simulink library "inp"  (Simulink 8.2)
settings: Solver=ode45  SolverMode=SingleTasking  StartTime=0.0  StopTime=10.0  RelTol=1e-3
undefined symbols (not defined in the file or in its folder): C_TICK_TIME_S, ENUM_FAULTSTATE_FAIL, ENUM_FAULTSTATE_INDETERMINATE, ENUM_FAULTSTATE_PASS, ENUM_KEYSWITCH_ON, FALSE, KINP_ABS1CANCommuOk_flg_ovrdflg, KINP_ABS1CANCommuOk_flg_ovrdval, KINP_ABS1CANPeriodTime_s, KINP_ABS1CANRolCntOk_flg_ovrdflg, KINP_ABS1CANRolCntOk_flg_ovrdval, KINP_ABS2CANChkSumOk_flg_ovrdflg, KINP_ABS2CANChkSumOk_flg_ovrdval, KINP_ABS2CANCommuOk_flg_ovrdflg, KINP_ABS2CANCommuOk_flg_ovrdval, KINP_ABS2CANPeriodTime_s, KINP_ABS2CANRolCntOk_flg_ovrdflg, KINP_ABS2CANRolCntOk_flg_ovrdval, KINP_ABS3CANCommuOk_flg_ovrdflg, KINP_ABS3CANCommuOk_flg_ovrdval, KINP_ABS4CANCommuOk_flg_ovrdflg, KINP_ABS4CANCommuOk_flg_ovrdval, KINP_ABSActive_flg_defval, KINP_ABSActive_flg_invalid, KINP_ABSActive_flg_ovrdflg, KINP_ABSActive_flg_ovrdval, KINP_ABSActive_flg_setvalid, KINP_ABSActive_flg_srvcflg, KINP_ABSActive_flg_srvcval, KINP_ABSCANAlive_flg_ovrdflg, KINP_ABSCANAlive_flg_ovrdval, KINP_ABSCANCommuOk_flg_ovrdflg, KINP_ABSCANCommuOk_flg_ovrdval, KINP_ABSCANValidOk_flg_ovrdflg, KINP_ABSCANValidOk_flg_ovrdval, KINP_ABSFault_flg_defval, KINP_ABSFault_flg_invalid, KINP_ABSFault_flg_ovrdflg, KINP_ABSFault_flg_ovrdval, KINP_ABSFault_flg_setvalid, KINP_ABSFault_flg_srvcflg, KINP_ABSFault_flg_srvcval, KINP_AC1CANCommuOk_flg_ovrdflg, KINP_AC1CANCommuOk_flg_ovrdval, KINP_ACCANAlive_flg_ovrdflg, KINP_ACCANAlive_flg_ovrdval, KINP_ACCANCommuOk_flg_ovrdflg, KINP_ACCANCommuOk_flg_ovrdval, KINP_ACCANValidOk_flg_ovrdflg, KINP_ACCANValidOk_flg_ovrdval, KINP_ACCCancelH_flg_defval, KINP_ACCCancelH_flg_invalid, KINP_ACCCancelH_flg_ovrdflg, KINP_ACCCancelH_flg_ovrdval, KINP_ACCCancelH_flg_setvalid, KINP_ACCCancelH_flg_srvcflg, KINP_ACCCancelH_flg_srvcval, KINP_AccelPedalPosn_pct_defval, KINP_AccelPedalPosn_pct_gain, KINP_AccelPedalPosn_pct_invalid ... (+1866)
libraries: inp x192, hcu_lib x34, simulink x33
(part 10 of 21)

== system inp/INP/EPM_signals/Cam_Obstacle_3 ==
  BHAL_outputs : Inport
  CANvalid_bus : Inport  Port=2
  Bus Creator : BusCreator  Inputs=40
  CANVBusSel{41..50} (10 alike) : BusSelector  OutputSignals=VINP_CANMsgValidEPM_flg.VINP_CANMsgValidEPM12_flg
  CANVBusSel{51..55} (5 alike) : BusSelector  OutputSignals=VINP_CANMsgValidEPM_flg.VINP_CANMsgValidEPM13_flg
  CANVBusSel{56..60} (5 alike) : BusSelector  OutputSignals=VINP_CANMsgValidEPM_flg.VINP_CANMsgValidEPM14_flg
  Constant{41..60} (20 alike) : Constant  Value=TRUE
  Conv{41,45..49,53..54} (8 alike) : DataTypeConversion  OutDataTypeStr=uint8
  Conv{42..44,51..52,56..59} (9 alike) : DataTypeConversion  OutDataTypeStr=single
  Conv50 : DataTypeConversion  OutDataTypeStr=boolean
  Conv55 : DataTypeConversion  OutDataTypeStr=boolean
  Conv60 : DataTypeConversion  OutDataTypeStr=boolean
  HalBusSel41 : BusSelector  OutputSignals=VHAL_CamObs3ID_num
  HalBusSel42 : BusSelector  OutputSignals=VHAL_CamObs3PosX_m
  HalBusSel43 : BusSelector  OutputSignals=VHAL_CamObs3PosY_m
  HalBusSel44 : BusSelector  OutputSignals=VHAL_CamObs3VelX_mps
  HalBusSel45 : BusSelector  OutputSignals=VHAL_CamObs3Type_enum
  HalBusSel46 : BusSelector  OutputSignals=VHAL_CamObs3Status_enum
  HalBusSel47 : BusSelector  OutputSignals=VHAL_CamObs3Valid_enum
  HalBusSel48 : BusSelector  OutputSignals=VHAL_CamObs3MoveInO_enum
  HalBusSel49 : BusSelector  OutputSignals=VHAL_CamObs3Blinker_enum
  HalBusSel50 : BusSelector  OutputSignals=VHAL_CamObs3BrakeLight_flg
  HalBusSel51 : BusSelector  OutputSignals=VHAL_CamObs3Length_m
  HalBusSel52 : BusSelector  OutputSignals=VHAL_CamObs3Width_m
  HalBusSel53 : BusSelector  OutputSignals=VHAL_CamObs3Age_cnt
  HalBusSel54 : BusSelector  OutputSignals=VHAL_CamObs3Lane_enum
  HalBusSel55 : BusSelector  OutputSignals=VHAL_CamObs3CIPV_flg
  HalBusSel56 : BusSelector  OutputSignals=VHAL_CamObs3VelY_mps
  HalBusSel57 : BusSelector  OutputSignals=VHAL_CamObs3AccX_mps2
  HalBusSel58 : BusSelector  OutputSignals=VHAL_CamObs3Ang_deg
  HalBusSel59 : BusSelector  OutputSignals=VHAL_CamObs3AngRate_degps
  HalBusSel60 : BusSelector  OutputSignals=VHAL_CamObs3Replaced_flg
  Term{41..60} (20 alike) : Terminator
  Cam_Obstacle_3 : Outport
  wires:
  CANVBusSel55/1 -> Term55/1  "<VINP_CANMsgValidEPM13_flg>"
  HalBusSel41/1 -> Conv41/1  "<VHAL_CamObs3ID_num>"
  [BHAL_outputs] -> HalBusSel41/1
  [CANvalid_bus] -> CANVBusSel41/1
  Constant41/1 -> Bus Creator/2  "VINP_CamObs3IDValid_flg_InHAL"
  Conv41/1 -> Bus Creator/1  "VINP_CamObs3ID_num_InHAL"
  CANVBusSel41/1 -> Term41/1  "<VINP_CANMsgValidEPM12_flg>"
  [CANvalid_bus] -> CANVBusSel42/1
  [BHAL_outputs] -> HalBusSel42/1
  HalBusSel42/1 -> Conv42/1  "<VHAL_CamObs3PosX_m>"
  Constant42/1 -> Bus Creator/4  "VINP_CamObs3PosXValid_flg_InHAL"
  Conv42/1 -> Bus Creator/3  "VINP_CamObs3PosX_m_InHAL"
  CANVBusSel42/1 -> Term42/1  "<VINP_CANMsgValidEPM12_flg>"
  HalBusSel43/1 -> Conv43/1  "<VHAL_CamObs3PosY_m>"
  [BHAL_outputs] -> HalBusSel43/1
  [CANvalid_bus] -> CANVBusSel43/1
  Constant43/1 -> Bus Creator/6  "VINP_CamObs3PosYValid_flg_InHAL"
  Conv43/1 -> Bus Creator/5  "VINP_CamObs3PosY_m_InHAL"
  CANVBusSel43/1 -> Term43/1  "<VINP_CANMsgValidEPM12_flg>"
  HalBusSel44/1 -> Conv44/1  "<VHAL_CamObs3VelX_mps>"
  [BHAL_outputs] -> HalBusSel44/1
  [CANvalid_bus] -> CANVBusSel44/1
  Constant44/1 -> Bus Creator/8  "VINP_CamObs3VelXValid_flg_InHAL"
  Conv44/1 -> Bus Creator/7  "VINP_CamObs3VelX_mps_InHAL"
  CANVBusSel44/1 -> Term44/1  "<VINP_CANMsgValidEPM12_flg>"
  HalBusSel45/1 -> Conv45/1  "<VHAL_CamObs3Type_enum>"
  [BHAL_outputs] -> HalBusSel45/1
  [CANvalid_bus] -> CANVBusSel45/1
  Constant45/1 -> Bus Creator/10  "VINP_CamObs3TypeValid_flg_InHAL"
  Conv45/1 -> Bus Creator/9  "VINP_CamObs3Type_enum_InHAL"
  CANVBusSel45/1 -> Term45/1  "<VINP_CANMsgValidEPM12_flg>"
  [BHAL_outputs] -> HalBusSel46/1
  HalBusSel46/1 -> Conv46/1  "<VHAL_CamObs3Status_enum>"
  Conv46/1 -> Bus Creator/11  "VINP_CamObs3Status_enum_InHAL"
  Constant46/1 -> Bus Creator/12  "VINP_CamObs3StatusValid_flg_InHAL"
  [CANvalid_bus] -> CANVBusSel46/1
  CANVBusSel46/1 -> Term46/1  "<VINP_CANMsgValidEPM12_flg>"
  [BHAL_outputs] -> HalBusSel47/1
  HalBusSel47/1 -> Conv47/1  "<VHAL_CamObs3Valid_enum>"
  [CANvalid_bus] -> CANVBusSel47/1
  Constant47/1 -> Bus Creator/14  "VINP_CamObs3ValidValid_flg_InHAL"
  Conv47/1 -> Bus Creator/13  "VINP_CamObs3Valid_enum_InHAL"
  CANVBusSel47/1 -> Term47/1  "<VINP_CANMsgValidEPM12_flg>"
  [BHAL_outputs] -> HalBusSel48/1
  HalBusSel48/1 -> Conv48/1  "<VHAL_CamObs3MoveInO_enum>"
  Conv48/1 -> Bus Creator/15  "VINP_CamObs3MoveInO_enum_InHAL"
  Constant48/1 -> Bus Creator/16  "VINP_CamObs3MoveInOValid_flg_InHAL"
  [CANvalid_bus] -> CANVBusSel48/1
  CANVBusSel48/1 -> Term48/1  "<VINP_CANMsgValidEPM12_flg>"
  [BHAL_outputs] -> HalBusSel49/1
  HalBusSel49/1 -> Conv49/1  "<VHAL_CamObs3Blinker_enum>"
  Conv49/1 -> Bus Creator/17  "VINP_CamObs3Blinker_enum_InHAL"
  Constant49/1 -> Bus Creator/18  "VINP_CamObs3BlinkerValid_flg_InHAL"
  [CANvalid_bus] -> CANVBusSel49/1
  CANVBusSel49/1 -> Term49/1  "<VINP_CANMsgValidEPM12_flg>"
  [BHAL_outputs] -> HalBusSel50/1
  HalBusSel50/1 -> Conv50/1  "<VHAL_CamObs3BrakeLight_flg>"
  Conv50/1 -> Bus Creator/19  "VINP_CamObs3BrakeLight_flg_InHAL"
  Constant50/1 -> Bus Creator/20  "VINP_CamObs3BrakeLightValid_flg_InHAL"
  [CANvalid_bus] -> CANVBusSel50/1
  CANVBusSel50/1 -> Term50/1  "<VINP_CANMsgValidEPM12_flg>"
  [BHAL_outputs] -> HalBusSel51/1
  [CANvalid_bus] -> CANVBusSel51/1
  HalBusSel51/1 -> Conv51/1  "<VHAL_CamObs3Length_m>"
  Constant51/1 -> Bus Creator/22  "VINP_CamObs3LengthValid_flg_InHAL"
  Conv51/1 -> Bus Creator/21  "VINP_CamObs3Length_m_InHAL"
  CANVBusSel51/1 -> Term51/1  "<VINP_CANMsgValidEPM13_flg>"
  [BHAL_outputs] -> HalBusSel52/1
  [CANvalid_bus] -> CANVBusSel52/1
  HalBusSel52/1 -> Conv52/1  "<VHAL_CamObs3Width_m>"
  Constant52/1 -> Bus Creator/24  "VINP_CamObs3WidthValid_flg_InHAL"
  Conv52/1 -> Bus Creator/23  "VINP_CamObs3Width_m_InHAL"
  CANVBusSel52/1 -> Term52/1  "<VINP_CANMsgValidEPM13_flg>"
  [BHAL_outputs] -> HalBusSel53/1
  [CANvalid_bus] -> CANVBusSel53/1
  HalBusSel53/1 -> Conv53/1  "<VHAL_CamObs3Age_cnt>"
  Constant53/1 -> Bus Creator/26  "VINP_CamObs3AgeValid_flg_InHAL"
  Conv53/1 -> Bus Creator/25  "VINP_CamObs3Age_cnt_InHAL"
  CANVBusSel53/1 -> Term53/1  "<VINP_CANMsgValidEPM13_flg>"
  HalBusSel54/1 -> Conv54/1  "<VHAL_CamObs3Lane_enum>"
  [BHAL_outputs] -> HalBusSel54/1
  [CANvalid_bus] -> CANVBusSel54/1
  Constant54/1 -> Bus Creator/28  "VINP_CamObs3LaneValid_flg_InHAL"
  Conv54/1 -> Bus Creator/27  "VINP_CamObs3Lane_enum_InHAL"
  CANVBusSel54/1 -> Term54/1  "<VINP_CANMsgValidEPM13_flg>"
  HalBusSel55/1 -> Conv55/1  "<VHAL_CamObs3CIPV_flg>"
  [BHAL_outputs] -> HalBusSel55/1
  [CANvalid_bus] -> CANVBusSel55/1
  Constant55/1 -> Bus Creator/30  "VINP_CamObs3CIPVValid_flg_InHAL"
  Conv55/1 -> Bus Creator/29  "VINP_CamObs3CIPV_flg_InHAL"
  Constant59/1 -> Bus Creator/38  "VINP_CamObs3AngRateValid_flg_InHAL"
  [CANvalid_bus] -> CANVBusSel59/1
  HalBusSel59/1 -> Conv59/1  "<VHAL_CamObs3AngRate_degps>"
  Conv59/1 -> Bus Creator/37  "VINP_CamObs3AngRate_degps_InHAL"
  [BHAL_outputs] -> HalBusSel59/1
  CANVBusSel58/1 -> Term58/1  "<VINP_CANMsgValidEPM14_flg>"
  Conv58/1 -> Bus Creator/35  "VINP_CamObs3Ang_deg_InHAL"
  Constant58/1 -> Bus Creator/36  "VINP_CamObs3AngValid_flg_InHAL"
  [CANvalid_bus] -> CANVBusSel58/1
  HalBusSel58/1 -> Conv58/1  "<VHAL_CamObs3Ang_deg>"
  [BHAL_outputs] -> HalBusSel60/1
  Conv60/1 -> Bus Creator/39  "VINP_CamObs3Replaced_flg_InHAL"
  Constant60/1 -> Bus Creator/40  "VINP_CamObs3ReplacedValid_flg_InHAL"
  HalBusSel60/1 -> Conv60/1  "<VHAL_CamObs3Replaced_flg>"
  CANVBusSel60/1 -> Term60/1  "<VINP_CANMsgValidEPM14_flg>"
  [CANvalid_bus] -> CANVBusSel60/1
  CANVBusSel59/1 -> Term59/1  "<VINP_CANMsgValidEPM14_flg>"
  CANVBusSel57/1 -> Term57/1  "<VINP_CANMsgValidEPM14_flg>"
  Constant57/1 -> Bus Creator/34  "VINP_CamObs3AccXValid_flg_InHAL"
  [BHAL_outputs] -> HalBusSel58/1
  [CANvalid_bus] -> CANVBusSel57/1
  Conv57/1 -> Bus Creator/33  "VINP_CamObs3AccX_mps2_InHAL"
  HalBusSel57/1 -> Conv57/1  "<VHAL_CamObs3AccX_mps2>"
  [BHAL_outputs] -> HalBusSel57/1
  Constant56/1 -> Bus Creator/32  "VINP_CamObs3VelYValid_flg_InHAL"
  CANVBusSel56/1 -> Term56/1  "<VINP_CANMsgValidEPM14_flg>"
  [CANvalid_bus] -> CANVBusSel56/1
  Conv56/1 -> Bus Creator/31  "VINP_CamObs3VelY_mps_InHAL"
  [BHAL_outputs] -> HalBusSel56/1
  HalBusSel56/1 -> Conv56/1  "<VHAL_CamObs3VelY_mps>"
  BHAL_outputs/1 -> [BHAL_outputs]
  CANvalid_bus/1 -> [CANvalid_bus]
  Bus Creator/1 -> Cam_Obstacle_3/1  "VINP_Obstacle3_BUS_InHAL"

== system inp/INP/EPM_signals/Cam_Obstacle_4 ==
  BHAL_outputs : Inport
  CANvalid_bus : Inport  Port=2
  Bus Creator : BusCreator  Inputs=40
  CANVBusSel{61..70} (10 alike) : BusSelector  OutputSignals=VINP_CANMsgValidEPM_flg.VINP_CANMsgValidEPM15_flg
  CANVBusSel{71..75} (5 alike) : BusSelector  OutputSignals=VINP_CANMsgValidEPM_flg.VINP_CANMsgValidEPM16_flg
  CANVBusSel{76..80} (5 alike) : BusSelector  OutputSignals=VINP_CANMsgValidEPM_flg.VINP_CANMsgValidEPM17_flg
  Constant{61..80} (20 alike) : Constant  Value=TRUE
  Conv{61,65..69,73..74} (8 alike) : DataTypeConversion  OutDataTypeStr=uint8
  Conv{62..64,71..72,76..79} (9 alike) : DataTypeConversion  OutDataTypeStr=single
  Conv70 : DataTypeConversion  OutDataTypeStr=boolean
  Conv75 : DataTypeConversion  OutDataTypeStr=boolean
  Conv80 : DataTypeConversion  OutDataTypeStr=boolean
  HalBusSel61 : BusSelector  OutputSignals=VHAL_CamObs4ID_num
  HalBusSel62 : BusSelector  OutputSignals=VHAL_CamObs4PosX_m
  HalBusSel63 : BusSelector  OutputSignals=VHAL_CamObs4PosY_m
  HalBusSel64 : BusSelector  OutputSignals=VHAL_CamObs4VelX_mps
  HalBusSel65 : BusSelector  OutputSignals=VHAL_CamObs4Type_enum
  HalBusSel66 : BusSelector  OutputSignals=VHAL_CamObs4Status_enum
  HalBusSel67 : BusSelector  OutputSignals=VHAL_CamObs4Valid_enum
  HalBusSel68 : BusSelector  OutputSignals=VHAL_CamObs4MoveInO_enum
  HalBusSel69 : BusSelector  OutputSignals=VHAL_CamObs4Blinker_enum
  HalBusSel70 : BusSelector  OutputSignals=VHAL_CamObs4BrakeLight_flg
  HalBusSel71 : BusSelector  OutputSignals=VHAL_CamObs4Length_m
  HalBusSel72 : BusSelector  OutputSignals=VHAL_CamObs4Width_m
  HalBusSel73 : BusSelector  OutputSignals=VHAL_CamObs4Age_cnt
  HalBusSel74 : BusSelector  OutputSignals=VHAL_CamObs4Lane_enum
  HalBusSel75 : BusSelector  OutputSignals=VHAL_CamObs4CIPV_flg
  HalBusSel76 : BusSelector  OutputSignals=VHAL_CamObs4VelY_mps
  HalBusSel77 : BusSelector  OutputSignals=VHAL_CamObs4AccX_mps2
  HalBusSel78 : BusSelector  OutputSignals=VHAL_CamObs4Ang_deg
  HalBusSel79 : BusSelector  OutputSignals=VHAL_CamObs4AngRate_degps
  HalBusSel80 : BusSelector  OutputSignals=VHAL_CamObs4Replaced_flg
  Term{61..80} (20 alike) : Terminator
  Cam_Obstacle_4 : Outport
  wires:
  Conv68/1 -> Bus Creator/15  "VINP_CamObs4MoveInO_enum_InHAL"
  HalBusSel68/1 -> Conv68/1  "<VHAL_CamObs4MoveInO_enum>"
  [BHAL_outputs] -> HalBusSel68/1
  CANVBusSel68/1 -> Term68/1  "<VINP_CANMsgValidEPM15_flg>"
  [BHAL_outputs] -> HalBusSel70/1
  HalBusSel71/1 -> Conv71/1  "<VHAL_CamObs4Length_m>"
  [CANvalid_bus] -> CANVBusSel72/1
  HalBusSel76/1 -> Conv76/1  "<VHAL_CamObs4VelY_mps>"
  [CANvalid_bus] -> CANVBusSel76/1
  Conv76/1 -> Bus Creator/31  "VINP_CamObs4VelY_mps_InHAL"
  Constant76/1 -> Bus Creator/32  "VINP_CamObs4VelYValid_flg_InHAL"
  CANVBusSel76/1 -> Term76/1  "<VINP_CANMsgValidEPM17_flg>"
  [BHAL_outputs] -> HalBusSel77/1
  HalBusSel77/1 -> Conv77/1  "<VHAL_CamObs4AccX_mps2>"
  [CANvalid_bus] -> CANVBusSel77/1
  Conv77/1 -> Bus Creator/33  "VINP_CamObs4AccX_mps2_InHAL"
  Constant77/1 -> Bus Creator/34  "VINP_CamObs4AccXValid_flg_InHAL"
  CANVBusSel77/1 -> Term77/1  "<VINP_CANMsgValidEPM17_flg>"
  [CANvalid_bus] -> CANVBusSel78/1
  [BHAL_outputs] -> HalBusSel78/1
  HalBusSel78/1 -> Conv78/1  "<VHAL_CamObs4Ang_deg>"
  Constant78/1 -> Bus Creator/36  "VINP_CamObs4AngValid_flg_InHAL"
  Conv78/1 -> Bus Creator/35  "VINP_CamObs4Ang_deg_InHAL"
  CANVBusSel78/1 -> Term78/1  "<VINP_CANMsgValidEPM17_flg>"
  [CANvalid_bus] -> CANVBusSel79/1
  [BHAL_outputs] -> HalBusSel79/1
  Conv79/1 -> Bus Creator/37  "VINP_CamObs4AngRate_degps_InHAL"
  HalBusSel79/1 -> Conv79/1  "<VHAL_CamObs4AngRate_degps>"
  [BHAL_outputs] -> HalBusSel80/1
  Constant79/1 -> Bus Creator/38  "VINP_CamObs4AngRateValid_flg_InHAL"
  CANVBusSel79/1 -> Term79/1  "<VINP_CANMsgValidEPM17_flg>"
  HalBusSel80/1 -> Conv80/1  "<VHAL_CamObs4Replaced_flg>"
  Conv80/1 -> Bus Creator/39  "VINP_CamObs4Replaced_flg_InHAL"
  [CANvalid_bus] -> CANVBusSel80/1
  Constant80/1 -> Bus Creator/40  "VINP_CamObs4ReplacedValid_flg_InHAL"
  CANVBusSel80/1 -> Term80/1  "<VINP_CANMsgValidEPM17_flg>"
  [CANvalid_bus] -> CANVBusSel68/1
  Constant68/1 -> Bus Creator/16  "VINP_CamObs4MoveInOValid_flg_InHAL"
  Conv69/1 -> Bus Creator/17  "VINP_CamObs4Blinker_enum_InHAL"
  [BHAL_outputs] -> HalBusSel69/1
  [CANvalid_bus] -> CANVBusSel69/1
  HalBusSel69/1 -> Conv69/1  "<VHAL_CamObs4Blinker_enum>"
  Constant69/1 -> Bus Creator/18  "VINP_CamObs4BlinkerValid_flg_InHAL"
  CANVBusSel69/1 -> Term69/1  "<VINP_CANMsgValidEPM15_flg>"
  HalBusSel70/1 -> Conv70/1  "<VHAL_CamObs4BrakeLight_flg>"
  Conv70/1 -> Bus Creator/19  "VINP_CamObs4BrakeLight_flg_InHAL"
  Constant70/1 -> Bus Creator/20  "VINP_CamObs4BrakeLightValid_flg_InHAL"
  [CANvalid_bus] -> CANVBusSel70/1
  CANVBusSel70/1 -> Term70/1  "<VINP_CANMsgValidEPM15_flg>"
  [BHAL_outputs] -> HalBusSel71/1
  Conv71/1 -> Bus Creator/21  "VINP_CamObs4Length_m_InHAL"
  [CANvalid_bus] -> CANVBusSel71/1
  CANVBusSel71/1 -> Term71/1  "<VINP_CANMsgValidEPM16_flg>"
  Constant71/1 -> Bus Creator/22  "VINP_CamObs4LengthValid_flg_InHAL"
  [BHAL_outputs] -> HalBusSel72/1
  HalBusSel72/1 -> Conv72/1  "<VHAL_CamObs4Width_m>"
  Constant72/1 -> Bus Creator/24  "VINP_CamObs4WidthValid_flg_InHAL"
  Conv72/1 -> Bus Creator/23  "VINP_CamObs4Width_m_InHAL"
  [BHAL_outputs] -> HalBusSel73/1
  CANVBusSel72/1 -> Term72/1  "<VINP_CANMsgValidEPM16_flg>"
  HalBusSel73/1 -> Conv73/1  "<VHAL_CamObs4Age_cnt>"
  [CANvalid_bus] -> CANVBusSel73/1
  Constant73/1 -> Bus Creator/26  "VINP_CamObs4AgeValid_flg_InHAL"
  Conv73/1 -> Bus Creator/25  "VINP_CamObs4Age_cnt_InHAL"
  [BHAL_outputs] -> HalBusSel74/1
  CANVBusSel73/1 -> Term73/1  "<VINP_CANMsgValidEPM16_flg>"
  HalBusSel74/1 -> Conv74/1  "<VHAL_CamObs4Lane_enum>"
  Conv74/1 -> Bus Creator/27  "VINP_CamObs4Lane_enum_InHAL"
  [CANvalid_bus] -> CANVBusSel74/1
  Constant74/1 -> Bus Creator/28  "VINP_CamObs4LaneValid_flg_InHAL"
  [BHAL_outputs] -> HalBusSel75/1
  CANVBusSel74/1 -> Term74/1  "<VINP_CANMsgValidEPM16_flg>"
  HalBusSel75/1 -> Conv75/1  "<VHAL_CamObs4CIPV_flg>"
  Constant75/1 -> Bus Creator/30  "VINP_CamObs4CIPVValid_flg_InHAL"
  [CANvalid_bus] -> CANVBusSel75/1
  Conv75/1 -> Bus Creator/29  "VINP_CamObs4CIPV_flg_InHAL"
  [BHAL_outputs] -> HalBusSel76/1
  CANVBusSel75/1 -> Term75/1  "<VINP_CANMsgValidEPM16_flg>"
  Constant61/1 -> Bus Creator/2  "VINP_CamObs4IDValid_flg_InHAL"
  [CANvalid_bus] -> CANVBusSel62/1
  Conv61/1 -> Bus Creator/1  "VINP_CamObs4ID_num_InHAL"
  CANVBusSel61/1 -> Term61/1  "<VINP_CANMsgValidEPM15_flg>"
  Constant62/1 -> Bus Creator/4  "VINP_CamObs4PosXValid_flg_InHAL"
  Conv62/1 -> Bus Creator/3  "VINP_CamObs4PosX_m_InHAL"
  HalBusSel62/1 -> Conv62/1  "<VHAL_CamObs4PosX_m>"
  [BHAL_outputs] -> HalBusSel63/1
  [BHAL_outputs] -> HalBusSel62/1
  [CANvalid_bus] -> CANVBusSel61/1
  CANVBusSel62/1 -> Term62/1  "<VINP_CANMsgValidEPM15_flg>"
  HalBusSel63/1 -> Conv63/1  "<VHAL_CamObs4PosY_m>"
  CANVBusSel63/1 -> Term63/1  "<VINP_CANMsgValidEPM15_flg>"
  Constant65/1 -> Bus Creator/10  "VINP_CamObs4TypeValid_flg_InHAL"
  [CANvalid_bus] -> CANVBusSel63/1
  HalBusSel61/1 -> Conv61/1  "<VHAL_CamObs4ID_num>"
  Constant63/1 -> Bus Creator/6  "VINP_CamObs4PosYValid_flg_InHAL"
  [CANvalid_bus] -> CANVBusSel64/1
  Conv63/1 -> Bus Creator/5  "VINP_CamObs4PosY_m_InHAL"
  [BHAL_outputs] -> HalBusSel65/1
  [BHAL_outputs] -> HalBusSel61/1
  CANVBusSel65/1 -> Term65/1  "<VINP_CANMsgValidEPM15_flg>"
  [BHAL_outputs] -> HalBusSel64/1
  HalBusSel64/1 -> Conv64/1  "<VHAL_CamObs4VelX_mps>"
  Conv64/1 -> Bus Creator/7  "VINP_CamObs4VelX_mps_InHAL"
  [CANvalid_bus] -> CANVBusSel65/1
  Constant64/1 -> Bus Creator/8  "VINP_CamObs4VelXValid_flg_InHAL"
  Conv65/1 -> Bus Creator/9  "VINP_CamObs4Type_enum_InHAL"
  HalBusSel65/1 -> Conv65/1  "<VHAL_CamObs4Type_enum>"
  CANVBusSel64/1 -> Term64/1  "<VINP_CANMsgValidEPM15_flg>"
  Conv66/1 -> Bus Creator/11  "VINP_CamObs4Status_enum_InHAL"
  [BHAL_outputs] -> HalBusSel66/1
  CANVBusSel66/1 -> Term66/1  "<VINP_CANMsgValidEPM15_flg>"
  [BHAL_outputs] -> HalBusSel67/1
  [CANvalid_bus] -> CANVBusSel67/1
  HalBusSel67/1 -> Conv67/1  "<VHAL_CamObs4Valid_enum>"
  Constant66/1 -> Bus Creator/12  "VINP_CamObs4StatusValid_flg_InHAL"
  Conv67/1 -> Bus Creator/13  "VINP_CamObs4Valid_enum_InHAL"
  [CANvalid_bus] -> CANVBusSel66/1
  HalBusSel66/1 -> Conv66/1  "<VHAL_CamObs4Status_enum>"
  CANVBusSel67/1 -> Term67/1  "<VINP_CANMsgValidEPM15_flg>"
  Constant67/1 -> Bus Creator/14  "VINP_CamObs4ValidValid_flg_InHAL"
  BHAL_outputs/1 -> [BHAL_outputs]
  CANvalid_bus/1 -> [CANvalid_bus]
  Bus Creator/1 -> Cam_Obstacle_4/1  "VINP_Obstacle4_BUS_InHAL"

== system inp/INP/EPM_signals/Cam_Obstacle_5 ==
  BHAL_outputs : Inport
  CANvalid_bus : Inport  Port=2
  Bus Creator : BusCreator  Inputs=40
  CANVBusSel{96..100} (5 alike) : BusSelector  OutputSignals=VINP_CANMsgValidEPM_flg.VINP_CANMsgValidEPM20_flg
  CANVBusSel{81..90} (10 alike) : BusSelector  OutputSignals=VINP_CANMsgValidEPM_flg.VINP_CANMsgValidEPM18_flg
  CANVBusSel{91..95} (5 alike) : BusSelector  OutputSignals=VINP_CANMsgValidEPM_flg.VINP_CANMsgValidEPM19_flg
  Constant{81..100} (20 alike) : Constant  Value=TRUE
  Conv100 : DataTypeConversion  OutDataTypeStr=boolean
  Conv{81,85..89,93..94} (8 alike) : DataTypeConversion  OutDataTypeStr=uint8
  Conv{82..84,91..92,96..99} (9 alike) : DataTypeConversion  OutDataTypeStr=single
  Conv90 : DataTypeConversion  OutDataTypeStr=boolean
  Conv95 : DataTypeConversion  OutDataTypeStr=boolean
  HalBusSel100 : BusSelector  OutputSignals=VHAL_CamObs5Replaced_flg
  HalBusSel81 : BusSelector  OutputSignals=VHAL_CamObs5ID_num
  HalBusSel82 : BusSelector  OutputSignals=VHAL_CamObs5PosX_m
  HalBusSel83 : BusSelector  OutputSignals=VHAL_CamObs5PosY_m
  HalBusSel84 : BusSelector  OutputSignals=VHAL_CamObs5VelX_mps
  HalBusSel85 : BusSelector  OutputSignals=VHAL_CamObs5Type_enum
  HalBusSel86 : BusSelector  OutputSignals=VHAL_CamObs5Status_enum
  HalBusSel87 : BusSelector  OutputSignals=VHAL_CamObs5Valid_enum
  HalBusSel88 : BusSelector  OutputSignals=VHAL_CamObs5MoveInO_enum
  HalBusSel89 : BusSelector  OutputSignals=VHAL_CamObs5Blinker_enum
  HalBusSel90 : BusSelector  OutputSignals=VHAL_CamObs5BrakeLight_flg
  HalBusSel91 : BusSelector  OutputSignals=VHAL_CamObs5Length_m
  HalBusSel92 : BusSelector  OutputSignals=VHAL_CamObs5Width_m
  HalBusSel93 : BusSelector  OutputSignals=VHAL_CamObs5Age_cnt
  HalBusSel94 : BusSelector  OutputSignals=VHAL_CamObs5Lane_enum
  HalBusSel95 : BusSelector  OutputSignals=VHAL_CamObs5CIPV_flg
  HalBusSel96 : BusSelector  OutputSignals=VHAL_CamObs5VelY_mps
  HalBusSel97 : BusSelector  OutputSignals=VHAL_CamObs5AccX_mps2
  HalBusSel98 : BusSelector  OutputSignals=VHAL_CamObs5Ang_deg
  HalBusSel99 : BusSelector  OutputSignals=VHAL_CamObs5AngRate_degps
  Term{81..100} (20 alike) : Terminator
  Cam_Obstacle_5 : Outport
  wires:
  [CANvalid_bus] -> CANVBusSel82/1
  [CANvalid_bus] -> CANVBusSel94/1
  HalBusSel93/1 -> Conv93/1  "<VHAL_CamObs5Age_cnt>"
  Conv95/1 -> Bus Creator/29  "VINP_CamObs5CIPV_flg_InHAL"
  Constant97/1 -> Bus Creator/34  "VINP_CamObs5AccXValid_flg_InHAL"
  CANVBusSel98/1 -> Term98/1  "<VINP_CANMsgValidEPM20_flg>"
  Constant83/1 -> Bus Creator/6  "VINP_CamObs5PosYValid_flg_InHAL"
  CANVBusSel84/1 -> Term84/1  "<VINP_CANMsgValidEPM18_flg>"
  [BHAL_outputs] -> HalBusSel86/1
  Constant81/1 -> Bus Creator/2  "VINP_CamObs5IDValid_flg_InHAL"
  [BHAL_outputs] -> HalBusSel81/1
  HalBusSel81/1 -> Conv81/1  "<VHAL_CamObs5ID_num>"
  [CANvalid_bus] -> CANVBusSel81/1
  Conv81/1 -> Bus Creator/1  "VINP_CamObs5ID_num_InHAL"
  [BHAL_outputs] -> HalBusSel82/1
  CANVBusSel81/1 -> Term81/1  "<VINP_CANMsgValidEPM18_flg>"
  HalBusSel82/1 -> Conv82/1  "<VHAL_CamObs5PosX_m>"
  Conv82/1 -> Bus Creator/3  "VINP_CamObs5PosX_m_InHAL"
  Constant82/1 -> Bus Creator/4  "VINP_CamObs5PosXValid_flg_InHAL"
  CANVBusSel82/1 -> Term82/1  "<VINP_CANMsgValidEPM18_flg>"
  [BHAL_outputs] -> HalBusSel83/1
  HalBusSel83/1 -> Conv83/1  "<VHAL_CamObs5PosY_m>"
  [CANvalid_bus] -> CANVBusSel83/1
  Conv83/1 -> Bus Creator/5  "VINP_CamObs5PosY_m_InHAL"
  CANVBusSel83/1 -> Term83/1  "<VINP_CANMsgValidEPM18_flg>"
  [BHAL_outputs] -> HalBusSel84/1
  HalBusSel84/1 -> Conv84/1  "<VHAL_CamObs5VelX_mps>"
  [CANvalid_bus] -> CANVBusSel84/1
  Conv84/1 -> Bus Creator/7  "VINP_CamObs5VelX_mps_InHAL"
  Constant84/1 -> Bus Creator/8  "VINP_CamObs5VelXValid_flg_InHAL"
  [BHAL_outputs] -> HalBusSel85/1
  HalBusSel85/1 -> Conv85/1  "<VHAL_CamObs5Type_enum>"
  [CANvalid_bus] -> CANVBusSel85/1
  Conv85/1 -> Bus Creator/9  "VINP_CamObs5Type_enum_InHAL"
  Constant85/1 -> Bus Creator/10  "VINP_CamObs5TypeValid_flg_InHAL"
  CANVBusSel85/1 -> Term85/1  "<VINP_CANMsgValidEPM18_flg>"
  Conv86/1 -> Bus Creator/11  "VINP_CamObs5Status_enum_InHAL"
  HalBusSel86/1 -> Conv86/1  "<VHAL_CamObs5Status_enum>"
  [CANvalid_bus] -> CANVBusSel86/1
  Constant86/1 -> Bus Creator/12  "VINP_CamObs5StatusValid_flg_InHAL"
  CANVBusSel86/1 -> Term86/1  "<VINP_CANMsgValidEPM18_flg>"
  [BHAL_outputs] -> HalBusSel87/1
  [CANvalid_bus] -> CANVBusSel87/1
  HalBusSel87/1 -> Conv87/1  "<VHAL_CamObs5Valid_enum>"
  Conv87/1 -> Bus Creator/13  "VINP_CamObs5Valid_enum_InHAL"
  Constant87/1 -> Bus Creator/14  "VINP_CamObs5ValidValid_flg_InHAL"
  CANVBusSel87/1 -> Term87/1  "<VINP_CANMsgValidEPM18_flg>"
  [BHAL_outputs] -> HalBusSel88/1
  Conv88/1 -> Bus Creator/15  "VINP_CamObs5MoveInO_enum_InHAL"
  HalBusSel88/1 -> Conv88/1  "<VHAL_CamObs5MoveInO_enum>"
  [CANvalid_bus] -> CANVBusSel88/1
  Constant88/1 -> Bus Creator/16  "VINP_CamObs5MoveInOValid_flg_InHAL"
  CANVBusSel88/1 -> Term88/1  "<VINP_CANMsgValidEPM18_flg>"
  [BHAL_outputs] -> HalBusSel89/1
  Conv89/1 -> Bus Creator/17  "VINP_CamObs5Blinker_enum_InHAL"
  HalBusSel89/1 -> Conv89/1  "<VHAL_CamObs5Blinker_enum>"
  [CANvalid_bus] -> CANVBusSel89/1
  Constant89/1 -> Bus Creator/18  "VINP_CamObs5BlinkerValid_flg_InHAL"
  CANVBusSel89/1 -> Term89/1  "<VINP_CANMsgValidEPM18_flg>"
  [BHAL_outputs] -> HalBusSel90/1
  Conv90/1 -> Bus Creator/19  "VINP_CamObs5BrakeLight_flg_InHAL"
  HalBusSel90/1 -> Conv90/1  "<VHAL_CamObs5BrakeLight_flg>"
  [CANvalid_bus] -> CANVBusSel90/1
  Constant90/1 -> Bus Creator/20  "VINP_CamObs5BrakeLightValid_flg_InHAL"
  CANVBusSel90/1 -> Term90/1  "<VINP_CANMsgValidEPM18_flg>"
  [BHAL_outputs] -> HalBusSel91/1
  HalBusSel91/1 -> Conv91/1  "<VHAL_CamObs5Length_m>"
  [CANvalid_bus] -> CANVBusSel91/1
  Conv91/1 -> Bus Creator/21  "VINP_CamObs5Length_m_InHAL"
  Constant91/1 -> Bus Creator/22  "VINP_CamObs5LengthValid_flg_InHAL"
  CANVBusSel91/1 -> Term91/1  "<VINP_CANMsgValidEPM19_flg>"
  [BHAL_outputs] -> HalBusSel92/1
  HalBusSel92/1 -> Conv92/1  "<VHAL_CamObs5Width_m>"
  [CANvalid_bus] -> CANVBusSel92/1
  Conv92/1 -> Bus Creator/23  "VINP_CamObs5Width_m_InHAL"
  Constant92/1 -> Bus Creator/24  "VINP_CamObs5WidthValid_flg_InHAL"
  CANVBusSel92/1 -> Term92/1  "<VINP_CANMsgValidEPM19_flg>"
  [BHAL_outputs] -> HalBusSel93/1
  [CANvalid_bus] -> CANVBusSel93/1
  Conv93/1 -> Bus Creator/25  "VINP_CamObs5Age_cnt_InHAL"
  Constant93/1 -> Bus Creator/26  "VINP_CamObs5AgeValid_flg_InHAL"
  CANVBusSel93/1 -> Term93/1  "<VINP_CANMsgValidEPM19_flg>"
  [BHAL_outputs] -> HalBusSel94/1
  HalBusSel94/1 -> Conv94/1  "<VHAL_CamObs5Lane_enum>"
  Conv94/1 -> Bus Creator/27  "VINP_CamObs5Lane_enum_InHAL"
  Constant94/1 -> Bus Creator/28  "VINP_CamObs5LaneValid_flg_InHAL"
  CANVBusSel94/1 -> Term94/1  "<VINP_CANMsgValidEPM19_flg>"
  [BHAL_outputs] -> HalBusSel95/1
  HalBusSel95/1 -> Conv95/1  "<VHAL_CamObs5CIPV_flg>"
  [CANvalid_bus] -> CANVBusSel95/1
  Constant95/1 -> Bus Creator/30  "VINP_CamObs5CIPVValid_flg_InHAL"
  CANVBusSel95/1 -> Term95/1  "<VINP_CANMsgValidEPM19_flg>"
  [BHAL_outputs] -> HalBusSel96/1
  HalBusSel96/1 -> Conv96/1  "<VHAL_CamObs5VelY_mps>"
  [CANvalid_bus] -> CANVBusSel96/1
  Conv96/1 -> Bus Creator/31  "VINP_CamObs5VelY_mps_InHAL"
  Constant96/1 -> Bus Creator/32  "VINP_CamObs5VelYValid_flg_InHAL"
  CANVBusSel96/1 -> Term96/1  "<VINP_CANMsgValidEPM20_flg>"
  [BHAL_outputs] -> HalBusSel97/1
  HalBusSel97/1 -> Conv97/1  "<VHAL_CamObs5AccX_mps2>"
  [CANvalid_bus] -> CANVBusSel97/1
  Conv97/1 -> Bus Creator/33  "VINP_CamObs5AccX_mps2_InHAL"
  CANVBusSel97/1 -> Term97/1  "<VINP_CANMsgValidEPM20_flg>"
  [BHAL_outputs] -> HalBusSel98/1
  HalBusSel98/1 -> Conv98/1  "<VHAL_CamObs5Ang_deg>"
  [CANvalid_bus] -> CANVBusSel98/1
  Conv98/1 -> Bus Creator/35  "VINP_CamObs5Ang_deg_InHAL"
  Constant98/1 -> Bus Creator/36  "VINP_CamObs5AngValid_flg_InHAL"
  [BHAL_outputs] -> HalBusSel99/1
  Conv99/1 -> Bus Creator/37  "VINP_CamObs5AngRate_degps_InHAL"
  HalBusSel99/1 -> Conv99/1  "<VHAL_CamObs5AngRate_degps>"
  [CANvalid_bus] -> CANVBusSel99/1
  Constant99/1 -> Bus Creator/38  "VINP_CamObs5AngRateValid_flg_InHAL"
  CANVBusSel99/1 -> Term99/1  "<VINP_CANMsgValidEPM20_flg>"
  [BHAL_outputs] -> HalBusSel100/1
  Conv100/1 -> Bus Creator/39  "VINP_CamObs5Replaced_flg_InHAL"
  HalBusSel100/1 -> Conv100/1  "<VHAL_CamObs5Replaced_flg>"
  [CANvalid_bus] -> CANVBusSel100/1
  Constant100/1 -> Bus Creator/40  "VINP_CamObs5ReplacedValid_flg_InHAL"
  CANVBusSel100/1 -> Term100/1  "<VINP_CANMsgValidEPM20_flg>"
  BHAL_outputs/1 -> [BHAL_outputs]
  CANvalid_bus/1 -> [CANvalid_bus]
  Bus Creator/1 -> Cam_Obstacle_5/1  "VINP_Obstacle5_BUS_InHAL"

== system inp/INP/EPM_signals/Cam_Obstacle_6 ==
  BHAL_outputs : Inport
  CANvalid_bus : Inport  Port=2
  Bus Creator : BusCreator  Inputs=40
  CANVBusSel{101..110} (10 alike) : BusSelector  OutputSignals=VINP_CANMsgValidEPM_flg.VINP_CANMsgValidEPM21_flg
  CANVBusSel{111..115} (5 alike) : BusSelector  OutputSignals=VINP_CANMsgValidEPM_flg.VINP_CANMsgValidEPM22_flg
  CANVBusSel{116..120} (5 alike) : BusSelector  OutputSignals=VINP_CANMsgValidEPM_flg.VINP_CANMsgValidEPM23_flg
  Constant{101..120} (20 alike) : Constant  Value=TRUE
  Conv{101,105..109,113..114} (8 alike) : DataTypeConversion  OutDataTypeStr=uint8
  Conv{102..104,111..112,116..119} (9 alike) : DataTypeConversion  OutDataTypeStr=single
  Conv110 : DataTypeConversion  OutDataTypeStr=boolean
  Conv115 : DataTypeConversion  OutDataTypeStr=boolean
  Conv120 : DataTypeConversion  OutDataTypeStr=boolean
  HalBusSel101 : BusSelector  OutputSignals=VHAL_CamObs6ID_num
  HalBusSel102 : BusSelector  OutputSignals=VHAL_CamObs6PosX_m
  HalBusSel103 : BusSelector  OutputSignals=VHAL_CamObs6PosY_m
  HalBusSel104 : BusSelector  OutputSignals=VHAL_CamObs6VelX_mps
  HalBusSel105 : BusSelector  OutputSignals=VHAL_CamObs6Type_enum
  HalBusSel106 : BusSelector  OutputSignals=VHAL_CamObs6Status_enum
  HalBusSel107 : BusSelector  OutputSignals=VHAL_CamObs6Valid_enum
  HalBusSel108 : BusSelector  OutputSignals=VHAL_CamObs6MoveInO_enum
  HalBusSel109 : BusSelector  OutputSignals=VHAL_CamObs6Blinker_enum
  HalBusSel110 : BusSelector  OutputSignals=VHAL_CamObs6BrakeLight_flg
  HalBusSel111 : BusSelector  OutputSignals=VHAL_CamObs6Length_m
  HalBusSel112 : BusSelector  OutputSignals=VHAL_CamObs6Width_m
  HalBusSel113 : BusSelector  OutputSignals=VHAL_CamObs6Age_cnt
  HalBusSel114 : BusSelector  OutputSignals=VHAL_CamObs6Lane_enum
  HalBusSel115 : BusSelector  OutputSignals=VHAL_CamObs6CIPV_flg
  HalBusSel116 : BusSelector  OutputSignals=VHAL_CamObs6VelY_mps
  HalBusSel117 : BusSelector  OutputSignals=VHAL_CamObs6AccX_mps2
  HalBusSel118 : BusSelector  OutputSignals=VHAL_CamObs6Ang_deg
  HalBusSel119 : BusSelector  OutputSignals=VHAL_CamObs6AngRate_degps
  HalBusSel120 : BusSelector  OutputSignals=VHAL_CamObs6Replaced_flg
  Term{101..120} (20 alike) : Terminator
  Cam_Obstacle_6 : Outport
  wires:
  [BHAL_outputs] -> HalBusSel101/1
  HalBusSel101/1 -> Conv101/1  "<VHAL_CamObs6ID_num>"
  [CANvalid_bus] -> CANVBusSel101/1
  Conv101/1 -> Bus Creator/1  "VINP_CamObs6ID_num_InHAL"
  Constant101/1 -> Bus Creator/2  "VINP_CamObs6IDValid_flg_InHAL"
  CANVBusSel101/1 -> Term101/1  "<VINP_CANMsgValidEPM21_flg>"
  [BHAL_outputs] -> HalBusSel102/1
  HalBusSel102/1 -> Conv102/1  "<VHAL_CamObs6PosX_m>"
  [CANvalid_bus] -> CANVBusSel102/1
  Conv102/1 -> Bus Creator/3  "VINP_CamObs6PosX_m_InHAL"
  Constant102/1 -> Bus Creator/4  "VINP_CamObs6PosXValid_flg_InHAL"
  CANVBusSel102/1 -> Term102/1  "<VINP_CANMsgValidEPM21_flg>"
  [BHAL_outputs] -> HalBusSel103/1
  HalBusSel103/1 -> Conv103/1  "<VHAL_CamObs6PosY_m>"
  [CANvalid_bus] -> CANVBusSel103/1
  Conv103/1 -> Bus Creator/5  "VINP_CamObs6PosY_m_InHAL"
  Constant103/1 -> Bus Creator/6  "VINP_CamObs6PosYValid_flg_InHAL"
  CANVBusSel103/1 -> Term103/1  "<VINP_CANMsgValidEPM21_flg>"
  [BHAL_outputs] -> HalBusSel104/1
  HalBusSel104/1 -> Conv104/1  "<VHAL_CamObs6VelX_mps>"
  [CANvalid_bus] -> CANVBusSel104/1
  Conv104/1 -> Bus Creator/7  "VINP_CamObs6VelX_mps_InHAL"
  Constant104/1 -> Bus Creator/8  "VINP_CamObs6VelXValid_flg_InHAL"
  CANVBusSel104/1 -> Term104/1  "<VINP_CANMsgValidEPM21_flg>"
  [BHAL_outputs] -> HalBusSel105/1
  HalBusSel105/1 -> Conv105/1  "<VHAL_CamObs6Type_enum>"
  [CANvalid_bus] -> CANVBusSel105/1
  Conv105/1 -> Bus Creator/9  "VINP_CamObs6Type_enum_InHAL"
  Constant105/1 -> Bus Creator/10  "VINP_CamObs6TypeValid_flg_InHAL"
  CANVBusSel105/1 -> Term105/1  "<VINP_CANMsgValidEPM21_flg>"
  [BHAL_outputs] -> HalBusSel106/1
  Conv106/1 -> Bus Creator/11  "VINP_CamObs6Status_enum_InHAL"
  HalBusSel106/1 -> Conv106/1  "<VHAL_CamObs6Status_enum>"
  [CANvalid_bus] -> CANVBusSel106/1
  Constant106/1 -> Bus Creator/12  "VINP_CamObs6StatusValid_flg_InHAL"
  CANVBusSel106/1 -> Term106/1  "<VINP_CANMsgValidEPM21_flg>"
  [BHAL_outputs] -> HalBusSel107/1
  [CANvalid_bus] -> CANVBusSel107/1
  HalBusSel107/1 -> Conv107/1  "<VHAL_CamObs6Valid_enum>"
  Conv107/1 -> Bus Creator/13  "VINP_CamObs6Valid_enum_InHAL"
  Constant107/1 -> Bus Creator/14  "VINP_CamObs6ValidValid_flg_InHAL"
  CANVBusSel107/1 -> Term107/1  "<VINP_CANMsgValidEPM21_flg>"
  [BHAL_outputs] -> HalBusSel108/1
  Conv108/1 -> Bus Creator/15  "VINP_CamObs6MoveInO_enum_InHAL"
  HalBusSel108/1 -> Conv108/1  "<VHAL_CamObs6MoveInO_enum>"
  [CANvalid_bus] -> CANVBusSel108/1
  Constant108/1 -> Bus Creator/16  "VINP_CamObs6MoveInOValid_flg_InHAL"
  CANVBusSel108/1 -> Term108/1  "<VINP_CANMsgValidEPM21_flg>"
  [BHAL_outputs] -> HalBusSel109/1
  Conv109/1 -> Bus Creator/17  "VINP_CamObs6Blinker_enum_InHAL"
  HalBusSel109/1 -> Conv109/1  "<VHAL_CamObs6Blinker_enum>"
  [CANvalid_bus] -> CANVBusSel109/1
  Constant109/1 -> Bus Creator/18  "VINP_CamObs6BlinkerValid_flg_InHAL"
  CANVBusSel109/1 -> Term109/1  "<VINP_CANMsgValidEPM21_flg>"
  [BHAL_outputs] -> HalBusSel110/1
  Conv110/1 -> Bus Creator/19  "VINP_CamObs6BrakeLight_flg_InHAL"
  HalBusSel110/1 -> Conv110/1  "<VHAL_CamObs6BrakeLight_flg>"
  [CANvalid_bus] -> CANVBusSel110/1
  Constant110/1 -> Bus Creator/20  "VINP_CamObs6BrakeLightValid_flg_InHAL"
  CANVBusSel110/1 -> Term110/1  "<VINP_CANMsgValidEPM21_flg>"
  [BHAL_outputs] -> HalBusSel111/1
  HalBusSel111/1 -> Conv111/1  "<VHAL_CamObs6Length_m>"
  [CANvalid_bus] -> CANVBusSel111/1
  Conv111/1 -> Bus Creator/21  "VINP_CamObs6Length_m_InHAL"
  Constant111/1 -> Bus Creator/22  "VINP_CamObs6LengthValid_flg_InHAL"
  CANVBusSel111/1 -> Term111/1  "<VINP_CANMsgValidEPM22_flg>"
  [BHAL_outputs] -> HalBusSel112/1
  HalBusSel112/1 -> Conv112/1  "<VHAL_CamObs6Width_m>"
  [CANvalid_bus] -> CANVBusSel112/1
  Conv112/1 -> Bus Creator/23  "VINP_CamObs6Width_m_InHAL"
  Constant112/1 -> Bus Creator/24  "VINP_CamObs6WidthValid_flg_InHAL"
  CANVBusSel112/1 -> Term112/1  "<VINP_CANMsgValidEPM22_flg>"
  [BHAL_outputs] -> HalBusSel113/1
  HalBusSel113/1 -> Conv113/1  "<VHAL_CamObs6Age_cnt>"
  [CANvalid_bus] -> CANVBusSel113/1
  Conv113/1 -> Bus Creator/25  "VINP_CamObs6Age_cnt_InHAL"
  Constant113/1 -> Bus Creator/26  "VINP_CamObs6AgeValid_flg_InHAL"
  CANVBusSel113/1 -> Term113/1  "<VINP_CANMsgValidEPM22_flg>"
  [BHAL_outputs] -> HalBusSel114/1
  HalBusSel114/1 -> Conv114/1  "<VHAL_CamObs6Lane_enum>"
  [CANvalid_bus] -> CANVBusSel114/1
  Conv114/1 -> Bus Creator/27  "VINP_CamObs6Lane_enum_InHAL"
  Constant114/1 -> Bus Creator/28  "VINP_CamObs6LaneValid_flg_InHAL"
  CANVBusSel114/1 -> Term114/1  "<VINP_CANMsgValidEPM22_flg>"
  [BHAL_outputs] -> HalBusSel115/1
  HalBusSel115/1 -> Conv115/1  "<VHAL_CamObs6CIPV_flg>"
  [CANvalid_bus] -> CANVBusSel115/1
  Conv115/1 -> Bus Creator/29  "VINP_CamObs6CIPV_flg_InHAL"
  Constant115/1 -> Bus Creator/30  "VINP_CamObs6CIPVValid_flg_InHAL"
  CANVBusSel115/1 -> Term115/1  "<VINP_CANMsgValidEPM22_flg>"
  [BHAL_outputs] -> HalBusSel116/1
  HalBusSel116/1 -> Conv116/1  "<VHAL_CamObs6VelY_mps>"
  [CANvalid_bus] -> CANVBusSel116/1
  Conv116/1 -> Bus Creator/31  "VINP_CamObs6VelY_mps_InHAL"
  Constant116/1 -> Bus Creator/32  "VINP_CamObs6VelYValid_flg_InHAL"
  CANVBusSel116/1 -> Term116/1  "<VINP_CANMsgValidEPM23_flg>"
  [BHAL_outputs] -> HalBusSel117/1
  HalBusSel117/1 -> Conv117/1  "<VHAL_CamObs6AccX_mps2>"
  [CANvalid_bus] -> CANVBusSel117/1
  Conv117/1 -> Bus Creator/33  "VINP_CamObs6AccX_mps2_InHAL"
  Constant117/1 -> Bus Creator/34  "VINP_CamObs6AccXValid_flg_InHAL"
  CANVBusSel117/1 -> Term117/1  "<VINP_CANMsgValidEPM23_flg>"
  [BHAL_outputs] -> HalBusSel118/1
  HalBusSel118/1 -> Conv118/1  "<VHAL_CamObs6Ang_deg>"
  [CANvalid_bus] -> CANVBusSel118/1
  Conv118/1 -> Bus Creator/35  "VINP_CamObs6Ang_deg_InHAL"
  Constant118/1 -> Bus Creator/36  "VINP_CamObs6AngValid_flg_InHAL"
  CANVBusSel118/1 -> Term118/1  "<VINP_CANMsgValidEPM23_flg>"
  [BHAL_outputs] -> HalBusSel119/1
  Conv119/1 -> Bus Creator/37  "VINP_CamObs6AngRate_degps_InHAL"
  HalBusSel119/1 -> Conv119/1  "<VHAL_CamObs6AngRate_degps>"
  [CANvalid_bus] -> CANVBusSel119/1
  Constant119/1 -> Bus Creator/38  "VINP_CamObs6AngRateValid_flg_InHAL"
  CANVBusSel119/1 -> Term119/1  "<VINP_CANMsgValidEPM23_flg>"
  [BHAL_outputs] -> HalBusSel120/1
  Conv120/1 -> Bus Creator/39  "VINP_CamObs6Replaced_flg_InHAL"
  HalBusSel120/1 -> Conv120/1  "<VHAL_CamObs6Replaced_flg>"
  [CANvalid_bus] -> CANVBusSel120/1
  Constant120/1 -> Bus Creator/40  "VINP_CamObs6ReplacedValid_flg_InHAL"
  CANVBusSel120/1 -> Term120/1  "<VINP_CANMsgValidEPM23_flg>"
  BHAL_outputs/1 -> [BHAL_outputs]
  CANvalid_bus/1 -> [CANvalid_bus]
  Bus Creator/1 -> Cam_Obstacle_6/1  "VINP_Obstacle6_BUS_InHAL"

== system inp/INP/EPM_signals/Cam_Obstacle_7 ==
  BHAL_outputs : Inport
  CANvalid_bus : Inport  Port=2
  Bus Creator : BusCreator  Inputs=40
  CANVBusSel{121..130} (10 alike) : BusSelector  OutputSignals=VINP_CANMsgValidEPM_flg.VINP_CANMsgValidEPM24_flg
  CANVBusSel{131..135} (5 alike) : BusSelector  OutputSignals=VINP_CANMsgValidEPM_flg.VINP_CANMsgValidEPM25_flg
  CANVBusSel{136..140} (5 alike) : BusSelector  OutputSignals=VINP_CANMsgValidEPM_flg.VINP_CANMsgValidEPM26_flg
  Constant{121..140} (20 alike) : Constant  Value=TRUE
  Conv{121,125..129,133..134} (8 alike) : DataTypeConversion  OutDataTypeStr=uint8
  Conv{122..124,131..132,136..139} (9 alike) : DataTypeConversion  OutDataTypeStr=single
  Conv130 : DataTypeConversion  OutDataTypeStr=boolean
  Conv135 : DataTypeConversion  OutDataTypeStr=boolean
  Conv140 : DataTypeConversion  OutDataTypeStr=boolean
  HalBusSel121 : BusSelector  OutputSignals=VHAL_CamObs7ID_num
  HalBusSel122 : BusSelector  OutputSignals=VHAL_CamObs7PosX_m
  HalBusSel123 : BusSelector  OutputSignals=VHAL_CamObs7PosY_m
  HalBusSel124 : BusSelector  OutputSignals=VHAL_CamObs7VelX_mps
  HalBusSel125 : BusSelector  OutputSignals=VHAL_CamObs7Type_enum
  HalBusSel126 : BusSelector  OutputSignals=VHAL_CamObs7Status_enum
  HalBusSel127 : BusSelector  OutputSignals=VHAL_CamObs7Valid_enum
  HalBusSel128 : BusSelector  OutputSignals=VHAL_CamObs7MoveInO_enum
  HalBusSel129 : BusSelector  OutputSignals=VHAL_CamObs7Blinker_enum
  HalBusSel130 : BusSelector  OutputSignals=VHAL_CamObs7BrakeLight_flg
  HalBusSel131 : BusSelector  OutputSignals=VHAL_CamObs7Length_m
  HalBusSel132 : BusSelector  OutputSignals=VHAL_CamObs7Width_m
  HalBusSel133 : BusSelector  OutputSignals=VHAL_CamObs7Age_cnt
  HalBusSel134 : BusSelector  OutputSignals=VHAL_CamObs7Lane_enum
  HalBusSel135 : BusSelector  OutputSignals=VHAL_CamObs7CIPV_flg
  HalBusSel136 : BusSelector  OutputSignals=VHAL_CamObs7VelY_mps
  HalBusSel137 : BusSelector  OutputSignals=VHAL_CamObs7AccX_mps2
  HalBusSel138 : BusSelector  OutputSignals=VHAL_CamObs7Ang_deg
  HalBusSel139 : BusSelector  OutputSignals=VHAL_CamObs7AngRate_degps
  HalBusSel140 : BusSelector  OutputSignals=VHAL_CamObs7Replaced_flg
  Term{121..140} (20 alike) : Terminator
  Cam_Obstacle_7 : Outport
  wires:
  [BHAL_outputs] -> HalBusSel121/1
  HalBusSel121/1 -> Conv121/1  "<VHAL_CamObs7ID_num>"
  [CANvalid_bus] -> CANVBusSel121/1
  Conv121/1 -> Bus Creator/1  "VINP_CamObs7ID_num_InHAL"
  Constant121/1 -> Bus Creator/2  "VINP_CamObs7IDValid_flg_InHAL"
  CANVBusSel121/1 -> Term121/1  "<VINP_CANMsgValidEPM24_flg>"
  [BHAL_outputs] -> HalBusSel122/1
  HalBusSel122/1 -> Conv122/1  "<VHAL_CamObs7PosX_m>"
  [CANvalid_bus] -> CANVBusSel122/1
  Conv122/1 -> Bus Creator/3  "VINP_CamObs7PosX_m_InHAL"
  Constant122/1 -> Bus Creator/4  "VINP_CamObs7PosXValid_flg_InHAL"
  CANVBusSel122/1 -> Term122/1  "<VINP_CANMsgValidEPM24_flg>"
  [BHAL_outputs] -> HalBusSel123/1
  HalBusSel123/1 -> Conv123/1  "<VHAL_CamObs7PosY_m>"
  [CANvalid_bus] -> CANVBusSel123/1
  Conv123/1 -> Bus Creator/5  "VINP_CamObs7PosY_m_InHAL"
  Constant123/1 -> Bus Creator/6  "VINP_CamObs7PosYValid_flg_InHAL"
  CANVBusSel123/1 -> Term123/1  "<VINP_CANMsgValidEPM24_flg>"
  [BHAL_outputs] -> HalBusSel124/1
  HalBusSel124/1 -> Conv124/1  "<VHAL_CamObs7VelX_mps>"
  [CANvalid_bus] -> CANVBusSel124/1
  Conv124/1 -> Bus Creator/7  "VINP_CamObs7VelX_mps_InHAL"
  Constant124/1 -> Bus Creator/8  "VINP_CamObs7VelXValid_flg_InHAL"
  CANVBusSel124/1 -> Term124/1  "<VINP_CANMsgValidEPM24_flg>"
  [BHAL_outputs] -> HalBusSel125/1
  HalBusSel125/1 -> Conv125/1  "<VHAL_CamObs7Type_enum>"
  [CANvalid_bus] -> CANVBusSel125/1
  Conv125/1 -> Bus Creator/9  "VINP_CamObs7Type_enum_InHAL"
  Constant125/1 -> Bus Creator/10  "VINP_CamObs7TypeValid_flg_InHAL"
  CANVBusSel125/1 -> Term125/1  "<VINP_CANMsgValidEPM24_flg>"
  [BHAL_outputs] -> HalBusSel126/1
  Conv126/1 -> Bus Creator/11  "VINP_CamObs7Status_enum_InHAL"
  HalBusSel126/1 -> Conv126/1  "<VHAL_CamObs7Status_enum>"
  [CANvalid_bus] -> CANVBusSel126/1
  Constant126/1 -> Bus Creator/12  "VINP_CamObs7StatusValid_flg_InHAL"
  CANVBusSel126/1 -> Term126/1  "<VINP_CANMsgValidEPM24_flg>"
  [BHAL_outputs] -> HalBusSel127/1
  [CANvalid_bus] -> CANVBusSel127/1
  HalBusSel127/1 -> Conv127/1  "<VHAL_CamObs7Valid_enum>"
  Conv127/1 -> Bus Creator/13  "VINP_CamObs7Valid_enum_InHAL"
  Constant127/1 -> Bus Creator/14  "VINP_CamObs7ValidValid_flg_InHAL"
  CANVBusSel127/1 -> Term127/1  "<VINP_CANMsgValidEPM24_flg>"
  [BHAL_outputs] -> HalBusSel128/1
  Conv128/1 -> Bus Creator/15  "VINP_CamObs7MoveInO_enum_InHAL"
  HalBusSel128/1 -> Conv128/1  "<VHAL_CamObs7MoveInO_enum>"
  [CANvalid_bus] -> CANVBusSel128/1
  Constant128/1 -> Bus Creator/16  "VINP_CamObs7MoveInOValid_flg_InHAL"
  CANVBusSel128/1 -> Term128/1  "<VINP_CANMsgValidEPM24_flg>"
  [BHAL_outputs] -> HalBusSel129/1
  Conv129/1 -> Bus Creator/17  "VINP_CamObs7Blinker_enum_InHAL"
  HalBusSel129/1 -> Conv129/1  "<VHAL_CamObs7Blinker_enum>"
  [CANvalid_bus] -> CANVBusSel129/1
  Constant129/1 -> Bus Creator/18  "VINP_CamObs7BlinkerValid_flg_InHAL"
  CANVBusSel129/1 -> Term129/1  "<VINP_CANMsgValidEPM24_flg>"
  [BHAL_outputs] -> HalBusSel130/1
  Conv130/1 -> Bus Creator/19  "VINP_CamObs7BrakeLight_flg_InHAL"
  HalBusSel130/1 -> Conv130/1  "<VHAL_CamObs7BrakeLight_flg>"
  [CANvalid_bus] -> CANVBusSel130/1
  Constant130/1 -> Bus Creator/20  "VINP_CamObs7BrakeLightValid_flg_InHAL"
  CANVBusSel130/1 -> Term130/1  "<VINP_CANMsgValidEPM24_flg>"
  [BHAL_outputs] -> HalBusSel131/1
  HalBusSel131/1 -> Conv131/1  "<VHAL_CamObs7Length_m>"
  [CANvalid_bus] -> CANVBusSel131/1
  Conv131/1 -> Bus Creator/21  "VINP_CamObs7Length_m_InHAL"
  Constant131/1 -> Bus Creator/22  "VINP_CamObs7LengthValid_flg_InHAL"
  CANVBusSel131/1 -> Term131/1  "<VINP_CANMsgValidEPM25_flg>"
  [BHAL_outputs] -> HalBusSel132/1
  HalBusSel132/1 -> Conv132/1  "<VHAL_CamObs7Width_m>"
  [CANvalid_bus] -> CANVBusSel132/1
  Conv132/1 -> Bus Creator/23  "VINP_CamObs7Width_m_InHAL"
  Constant132/1 -> Bus Creator/24  "VINP_CamObs7WidthValid_flg_InHAL"
  CANVBusSel132/1 -> Term132/1  "<VINP_CANMsgValidEPM25_flg>"
  [BHAL_outputs] -> HalBusSel133/1
  HalBusSel133/1 -> Conv133/1  "<VHAL_CamObs7Age_cnt>"
  [CANvalid_bus] -> CANVBusSel133/1
  Conv133/1 -> Bus Creator/25  "VINP_CamObs7Age_cnt_InHAL"
  Constant133/1 -> Bus Creator/26  "VINP_CamObs7AgeValid_flg_InHAL"
  CANVBusSel133/1 -> Term133/1  "<VINP_CANMsgValidEPM25_flg>"
  [BHAL_outputs] -> HalBusSel134/1
  HalBusSel134/1 -> Conv134/1  "<VHAL_CamObs7Lane_enum>"
  [CANvalid_bus] -> CANVBusSel134/1
  Conv134/1 -> Bus Creator/27  "VINP_CamObs7Lane_enum_InHAL"
  Constant134/1 -> Bus Creator/28  "VINP_CamObs7LaneValid_flg_InHAL"
  CANVBusSel134/1 -> Term134/1  "<VINP_CANMsgValidEPM25_flg>"
  [BHAL_outputs] -> HalBusSel135/1
  HalBusSel135/1 -> Conv135/1  "<VHAL_CamObs7CIPV_flg>"
  [CANvalid_bus] -> CANVBusSel135/1
  Conv135/1 -> Bus Creator/29  "VINP_CamObs7CIPV_flg_InHAL"
  Constant135/1 -> Bus Creator/30  "VINP_CamObs7CIPVValid_flg_InHAL"
  CANVBusSel135/1 -> Term135/1  "<VINP_CANMsgValidEPM25_flg>"
  [BHAL_outputs] -> HalBusSel136/1
  HalBusSel136/1 -> Conv136/1  "<VHAL_CamObs7VelY_mps>"
  [CANvalid_bus] -> CANVBusSel136/1
  Conv136/1 -> Bus Creator/31  "VINP_CamObs7VelY_mps_InHAL"
  Constant136/1 -> Bus Creator/32  "VINP_CamObs7VelYValid_flg_InHAL"
  CANVBusSel136/1 -> Term136/1  "<VINP_CANMsgValidEPM26_flg>"
  [BHAL_outputs] -> HalBusSel137/1
  HalBusSel137/1 -> Conv137/1  "<VHAL_CamObs7AccX_mps2>"
  [CANvalid_bus] -> CANVBusSel137/1
  Conv137/1 -> Bus Creator/33  "VINP_CamObs7AccX_mps2_InHAL"
  Constant137/1 -> Bus Creator/34  "VINP_CamObs7AccXValid_flg_InHAL"
  CANVBusSel137/1 -> Term137/1  "<VINP_CANMsgValidEPM26_flg>"
  [BHAL_outputs] -> HalBusSel138/1
  HalBusSel138/1 -> Conv138/1  "<VHAL_CamObs7Ang_deg>"
  [CANvalid_bus] -> CANVBusSel138/1
  Conv138/1 -> Bus Creator/35  "VINP_CamObs7Ang_deg_InHAL"
  Constant138/1 -> Bus Creator/36  "VINP_CamObs7AngValid_flg_InHAL"
  CANVBusSel138/1 -> Term138/1  "<VINP_CANMsgValidEPM26_flg>"
  [BHAL_outputs] -> HalBusSel139/1
  Conv139/1 -> Bus Creator/37  "VINP_CamObs7AngRate_degps_InHAL"
  HalBusSel139/1 -> Conv139/1  "<VHAL_CamObs7AngRate_degps>"
  [CANvalid_bus] -> CANVBusSel139/1
  Constant139/1 -> Bus Creator/38  "VINP_CamObs7AngRateValid_flg_InHAL"
  CANVBusSel139/1 -> Term139/1  "<VINP_CANMsgValidEPM26_flg>"
  [BHAL_outputs] -> HalBusSel140/1
  Conv140/1 -> Bus Creator/39  "VINP_CamObs7Replaced_flg_InHAL"
  HalBusSel140/1 -> Conv140/1  "<VHAL_CamObs7Replaced_flg>"
  [CANvalid_bus] -> CANVBusSel140/1
  Constant140/1 -> Bus Creator/40  "VINP_CamObs7ReplacedValid_flg_InHAL"
  CANVBusSel140/1 -> Term140/1  "<VINP_CANMsgValidEPM26_flg>"
  BHAL_outputs/1 -> [BHAL_outputs]
  CANvalid_bus/1 -> [CANvalid_bus]
  Bus Creator/1 -> Cam_Obstacle_7/1  "VINP_Obstacle7_BUS_InHAL"
